simulink model "os_polyphase_synth_4096c"  (R2021a) (continued)
== system os_polyphase_synth_4096c/pfb_fir/pol1_in1_tap23 == (continued)
      | C-C-B*(D+A)
      | C-C-B*(D+A)-CARRYIN
      | C-C-B*(D+ACIN)
      | C-C-B*(D+ACIN)-CARRYIN
      | C-C-B*(D-A)
      | C-C-B*(D-A)-CARRYIN
      | C-C-B*(D-ACIN)
      | C-C-B*(D-ACIN)-CARRYIN
      | C-C-B*A
      | C-C-B*A-CARRYIN
      | C-C-B*ACIN
      | C-C-B*ACIN-CARRYIN
      | C-C-B*D
      | C-C-B*D-CARRYIN
      | C-C-BCIN*(A+D)
      | C-C-BCIN*(A+D)-CARRYIN
      | C-C-BCIN*(ACIN+D)
      | C-C-BCIN*(ACIN+D)-CARRYIN
      | C-C-BCIN*(D+A)
      | C-C-BCIN*(D+A)-CARRYIN
      | C-C-BCIN*(D+ACIN)
      | C-C-BCIN*(D+ACIN)-CARRYIN
      | C-C-BCIN*(D-A)
      | C-C-BCIN*(D-A)-CARRYIN
      | C-C-BCIN*(D-ACIN)
      | C-C-BCIN*(D-ACIN)-CARRYIN
      | C-C-BCIN*A
      | C-C-BCIN*A-CARRYIN
      | C-C-BCIN*ACIN
      | C-C-BCIN*ACIN-CARRYIN
      | C-C-BCIN*D
      | C-C-BCIN*D-CARRYIN
      | C-C-C
      | C-C-C-CARRYIN
      | C-C-C-CONCAT
      | C-C-C-CONCAT-CARRYIN
      | C-C-C-P
      | C-C-C-P-CARRYIN
      | C-C-CARRYCASCIN
      | C-C-CARRYIN
      | C-C-CONCAT
      | C-C-CONCAT-C
      | C-C-CONCAT-C-CARRYIN
      | C-C-CONCAT-CARRYIN
      | C-C-CONCAT-P
      | C-C-CONCAT-P-CARRYIN
      | C-C-D
      | C-C-D*B
      | C-C-D*B-CARRYIN
      | C-C-D*BCIN
      | C-C-D*BCIN-CARRYIN
      | C-C-D*D
      | C-C-D*D-CARRYIN
      | C-C-D-CARRYIN
      | C-C-P
      | C-C-P-C
      | C-C-P-C-CARRYIN
      | C-C-P-CARRYIN
      | C-C-P-CONCAT
      | C-C-P-CONCAT-CARRYIN
      | C-C-P-P
      | C-C-P-P-CARRYIN
      | C-CARRYCASCIN
      | C-CARRYIN
      | C-CONCAT
      | C-CONCAT-C
      | C-CONCAT-C-C
      | C-CONCAT-C-C-CARRYIN
      | C-CONCAT-C-CARRYIN
      | C-CONCAT-C-P
      | C-CONCAT-C-P-CARRYIN
      | C-CONCAT-CARRYCASCIN
      | C-CONCAT-CARRYIN
      | C-CONCAT-P
      | C-CONCAT-P-C
      | C-CONCAT-P-C-CARRYIN
      | C-CONCAT-P-CARRYIN
      | C-D
      | C-D*B
      | C-D*B-C
      | C-D*B-C-CARRYIN
      | C-D*B-CARRYIN
      | C-D*B-P
      | C-D*B-P-CARRYIN
      | C-D*BCIN
      | C-D*BCIN-C
      | C-D*BCIN-C-CARRYIN
      | C-D*BCIN-CARRYIN
      | C-D*BCIN-P
      | C-D*BCIN-P-CARRYIN
      | C-D*D
      | C-D*D-C
      | C-D*D-C-CARRYIN
      | C-D*D-CARRYIN
      | C-D*D-P
      | C-D*D-P-CARRYIN
      | C-D-C
      | C-D-C-CARRYIN
      | C-D-CARRYIN
      | C-D-P
      | C-D-P-CARRYIN
      | C-P
      | C-P-(A+D)
      | C-P-(A+D)*(A+D)
      | C-P-(A+D)*(A+D)-CARRYIN
      | C-P-(A+D)*B
      | C-P-(A+D)*B-CARRYIN
      | C-P-(A+D)*BCIN
      | C-P-(A+D)*BCIN-CARRYIN
      | C-P-(A+D)-CARRYIN
      | C-P-(ACIN+D)
      | C-P-(ACIN+D)*(ACIN+D)
      | C-P-(ACIN+D)*(ACIN+D)-CARRYIN
      | C-P-(ACIN+D)*B
      | C-P-(ACIN+D)*B-CARRYIN
      | C-P-(ACIN+D)*BCIN
      | C-P-(ACIN+D)*BCIN-CARRYIN
      | C-P-(ACIN+D)-CARRYIN
      | C-P-(D+A)
      | C-P-(D+A)*(D+A)
      | C-P-(D+A)*(D+A)-CARRYIN
      | C-P-(D+A)*B
      | C-P-(D+A)*B-CARRYIN
      | C-P-(D+A)*BCIN
      | C-P-(D+A)*BCIN-CARRYIN
      | C-P-(D+A)-CARRYIN
      | C-P-(D+ACIN)
      | C-P-(D+ACIN)*(D+ACIN)
      | C-P-(D+ACIN)*(D+ACIN)-CARRYIN
      | C-P-(D+ACIN)*B
      | C-P-(D+ACIN)*B-CARRYIN
      | C-P-(D+ACIN)*BCIN
      | C-P-(D+ACIN)*BCIN-CARRYIN
      | C-P-(D+ACIN)-CARRYIN
      | C-P-(D-A)
      | C-P-(D-A)*(D-A)
      | C-P-(D-A)*(D-A)-CARRYIN
      | C-P-(D-A)*B
      | C-P-(D-A)*B-CARRYIN
      | C-P-(D-A)*BCIN
      | C-P-(D-A)*BCIN-CARRYIN
      | C-P-(D-A)-CARRYIN
      | C-P-(D-ACIN)
      | C-P-(D-ACIN)*(D-ACIN)
      | C-P-(D-ACIN)*(D-ACIN)-CARRYIN
      | C-P-(D-ACIN)*B
      | C-P-(D-ACIN)*B-CARRYIN
      | C-P-(D-ACIN)*BCIN
      | C-P-(D-ACIN)*BCIN-CARRYIN
      | C-P-(D-ACIN)-CARRYIN
      | C-P-A
      | C-P-A*A
      | C-P-A*A-CARRYIN
      | C-P-A*B
      | C-P-A*B-CARRYIN
      | C-P-A*BCIN
      | C-P-A*BCIN-CARRYIN
      | C-P-A-CARRYIN
      | C-P-ACIN
      | C-P-ACIN*ACIN
      | C-P-ACIN*ACIN-CARRYIN
      | C-P-ACIN*B
      | C-P-ACIN*B-CARRYIN
      | C-P-ACIN*BCIN
      | C-P-ACIN*BCIN-CARRYIN
      | C-P-ACIN-CARRYIN
      | C-P-B*(A+D)
      | C-P-B*(A+D)-CARRYIN
      | C-P-B*(ACIN+D)
      | C-P-B*(ACIN+D)-CARRYIN
      | C-P-B*(D+A)
      | C-P-B*(D+A)-CARRYIN
      | C-P-B*(D+ACIN)
      | C-P-B*(D+ACIN)-CARRYIN
      | C-P-B*(D-A)
      | C-P-B*(D-A)-CARRYIN
      | C-P-B*(D-ACIN)
      | C-P-B*(D-ACIN)-CARRYIN
      | C-P-B*A
      | C-P-B*A-CARRYIN
      | C-P-B*ACIN
      | C-P-B*ACIN-CARRYIN
      | C-P-B*D
      | C-P-B*D-CARRYIN
      | C-P-BCIN*(A+D)
      | C-P-BCIN*(A+D)-CARRYIN
      | C-P-BCIN*(ACIN+D)
      | C-P-BCIN*(ACIN+D)-CARRYIN
      | C-P-BCIN*(D+A)
      | C-P-BCIN*(D+A)-CARRYIN
      | C-P-BCIN*(D+ACIN)
      | C-P-BCIN*(D+ACIN)-CARRYIN
      | C-P-BCIN*(D-A)
      | C-P-BCIN*(D-A)-CARRYIN
      | C-P-BCIN*(D-ACIN)
      | C-P-BCIN*(D-ACIN)-CARRYIN
      | C-P-BCIN*A
      | C-P-BCIN*A-CARRYIN
      | C-P-BCIN*ACIN
      | C-P-BCIN*ACIN-CARRYIN
      | C-P-BCIN*D
      | C-P-BCIN*D-CARRYIN
      | C-P-C
      | C-P-C-C
      | C-P-C-C-CARRYIN
      | C-P-C-CARRYIN
      | C-P-C-CONCAT
      | C-P-C-CONCAT-CARRYIN
      | C-P-C-P
      | C-P-C-P-CARRYIN
      | C-P-CARRYCASCIN
      | C-P-CARRYIN
      | C-P-CONCAT
      | C-P-CONCAT-C
      | C-P-CONCAT-C-CARRYIN
      | C-P-CONCAT-CARRYIN
      | C-P-D
      | C-P-D*B
      | C-P-D*B-CARRYIN
      | C-P-D*BCIN
      | C-P-D*BCIN-CARRYIN
      | C-P-D*D
      | C-P-D*D-CARRYIN
      | C-P-D-CARRYIN
      | C-P-P
      | C-P-P-C
      | C-P-P-C-CARRYIN
      | C-P-P-CARRYIN
      | C-P>>17
      | C-PCIN
      | C-PCIN>>17
      | CARRYIN
      | CONCAT
      | CONCAT+C
      | CONCAT+C+C
      | CONCAT+C+C+C
      | CONCAT+C+C+C+CARRYIN
      | CONCAT+C+C+CARRYIN
      | CONCAT+C+C+P
      | CONCAT+C+C+P+CARRYIN
      | CONCAT+C+C+P>>17
      | CONCAT+C+C+P>>17+CARRYIN
      | CONCAT+C+C+PCIN
      | CONCAT+C+C+PCIN+CARRYIN
      | CONCAT+C+C+PCIN>>17
      | CONCAT+C+C+PCIN>>17+CARRYIN
      | CONCAT+C+C-C
      | CONCAT+C+C-P
      | CONCAT+C+C-P>>17
      | CONCAT+C+C-PCIN
      | CONCAT+C+C-PCIN>>17
      | CONCAT+C+CARRYCASCIN
      | CONCAT+C+CARRYIN
      | CONCAT+C+P
      | CONCAT+C+P+C
      | CONCAT+C+P+C+CARRYIN
      | CONCAT+C+P+CARRYIN
      | CONCAT+C+P+P
      | CONCAT+C+P+P+CARRYIN
      | CONCAT+C+P+P>>17
      | CONCAT+C+P+P>>17+CARRYIN
      | CONCAT+C+P+PCIN
      | CONCAT+C+P+PCIN+CARRYIN
      | CONCAT+C+P+PCIN>>17
      | CONCAT+C+P+PCIN>>17+CARRYIN
      | CONCAT+C+P-C
      | CONCAT+C+P-P
      | CONCAT+C+P-P>>17
      | CONCAT+C+P-PCIN
      | CONCAT+C+P-PCIN>>17
      | CONCAT+C+P>>17
      | CONCAT+C+P>>17+C
      | CONCAT+C+P>>17+C+CARRYIN
      | CONCAT+C+P>>17+CARRYIN
      | CONCAT+C+P>>17+P
      | CONCAT+C+P>>17+P+CARRYIN
      | CONCAT+C+PCIN
      | CONCAT+C+PCIN+C
      | CONCAT+C+PCIN+C+CARRYIN
      | CONCAT+C+PCIN+CARRYIN
      | CONCAT+C+PCIN+P
      | CONCAT+C+PCIN+P+CARRYIN
      | CONCAT+C+PCIN>>17
      | CONCAT+C+PCIN>>17+C
      | CONCAT+C+PCIN>>17+C+CARRYIN
      | CONCAT+C+PCIN>>17+CARRYIN
      | CONCAT+C+PCIN>>17+P
      | CONCAT+C+PCIN>>17+P+CARRYIN
      | CONCAT+C-C
      | CONCAT+C-P
      | CONCAT+C-P>>17
      | CONCAT+C-PCIN
      | CONCAT+C-PCIN>>17
      | CONCAT+CARRYCASCIN
      | CONCAT+CARRYIN
      | CONCAT+P
      | CONCAT+P+C
      | CONCAT+P+C+C
      | CONCAT+P+C+C+CARRYIN
      | CONCAT+P+C+CARRYIN
      | CONCAT+P+C+P
      | CONCAT+P+C+P+CARRYIN
      | CONCAT+P+C+P>>17
      | CONCAT+P+C+P>>17+CARRYIN
      | CONCAT+P+C+PCIN
      | CONCAT+P+C+PCIN+CARRYIN
      | CONCAT+P+C+PCIN>>17
      | CONCAT+P+C+PCIN>>17+CARRYIN
      | CONCAT+P+C-C
      | CONCAT+P+C-P
      | CONCAT+P+C-P>>17
      | CONCAT+P+C-PCIN
      | CONCAT+P+C-PCIN>>17
      | CONCAT+P+CARRYCASCIN
      | CONCAT+P+CARRYIN
      | CONCAT+P+P
      | CONCAT+P+P+C
      | CONCAT+P+P+C+CARRYIN
      | CONCAT+P+P+CARRYIN
      | CONCAT+P+P>>17
      | CONCAT+P+P>>17+C
      | CONCAT+P+P>>17+C+CARRYIN
      | CONCAT+P+P>>17+CARRYIN
      | CONCAT+P+PCIN
      | CONCAT+P+PCIN+C
      | CONCAT+P+PCIN+C+CARRYIN
      | CONCAT+P+PCIN+CARRYIN
      | CONCAT+P+PCIN>>17
      | CONCAT+P+PCIN>>17+C
      | CONCAT+P+PCIN>>17+C+CARRYIN
      | CONCAT+P+PCIN>>17+CARRYIN
      | CONCAT+P-C
      | CONCAT+P-P
      | CONCAT+P-P>>17
      | CONCAT+P-PCIN
      | CONCAT+P-PCIN>>17
      | CONCAT+P>>17
      | CONCAT+P>>17+C
      | CONCAT+P>>17+C+C
      | CONCAT+P>>17+C+C+CARRYIN
      | CONCAT+P>>17+C+CARRYIN
      | CONCAT+P>>17+C+P
      | CONCAT+P>>17+C+P+CARRYIN
      | CONCAT+P>>17+CARRYIN
      | CONCAT+P>>17+P
      | CONCAT+P>>17+P+C
      | CONCAT+P>>17+P+C+CARRYIN
      | CONCAT+P>>17+P+CARRYIN
      | CONCAT+PCIN
      | CONCAT+PCIN+C
      | CONCAT+PCIN+C+C
      | CONCAT+PCIN+C+C+CARRYIN
      | CONCAT+PCIN+C+CARRYIN
      | CONCAT+PCIN+C+P
      | CONCAT+PCIN+C+P+CARRYIN
      | CONCAT+PCIN+CARRYIN
      | CONCAT+PCIN+P
      | CONCAT+PCIN+P+C
      | CONCAT+PCIN+P+C+CARRYIN
      | CONCAT+PCIN+P+CARRYIN
      | CONCAT+PCIN>>17
      | CONCAT+PCIN>>17+C
      | CONCAT+PCIN>>17+C+C
      | CONCAT+PCIN>>17+C+C+CARRYIN
      | CONCAT+PCIN>>17+C+CARRYIN
      | CONCAT+PCIN>>17+C+P
      | CONCAT+PCIN>>17+C+P+CARRYIN
      | CONCAT+PCIN>>17+CARRYIN
      | CONCAT+PCIN>>17+P
      | CONCAT+PCIN>>17+P+C
      | CONCAT+PCIN>>17+P+C+CARRYIN
      | CONCAT+PCIN>>17+P+CARRYIN
      | CONCAT-C
      | CONCAT-P
      | CONCAT-P>>17
      | CONCAT-PCIN
      | CONCAT-PCIN>>17
      | D
      | D*B
      | D*B+C
      | D*B+C+C
      | D*B+C+C+CARRYIN
      | D*B+C+CARRYIN
      | D*B+C+P
      | D*B+C+P+CARRYIN
      | D*B+C+P>>17
      | D*B+C+P>>17+CARRYIN
      | D*B+C+PCIN
      | D*B+C+PCIN+CARRYIN
      | D*B+C+PCIN>>17
      | D*B+C+PCIN>>17+CARRYIN
      | D*B+C-C
      | D*B+C-P
      | D*B+C-P>>17
      | D*B+C-PCIN
      | D*B+C-PCIN>>17
      | D*B+CARRYIN
      | D*B+P
      | D*B+P+C
      | D*B+P+C+CARRYIN
      | D*B+P+CARRYIN
      | D*B+P+P
      | D*B+P+P+CARRYIN
      | D*B+P+P>>17
      | D*B+P+P>>17+CARRYIN
      | D*B+P+PCIN
      | D*B+P+PCIN+CARRYIN
      | D*B+P+PCIN>>17
      | D*B+P+PCIN>>17+CARRYIN
      | D*B+P-C
      | D*B+P-P
      | D*B+P-P>>17
      | D*B+P-PCIN
      | D*B+P-PCIN>>17
      | D*B+P>>17
      | D*B+P>>17+C
      | D*B+P>>17+C+CARRYIN
      | D*B+P>>17+CARRYIN
      | D*B+P>>17+P
      | D*B+P>>17+P+CARRYIN
      | D*B+PCIN
      | D*B+PCIN+C
      | D*B+PCIN+C+CARRYIN
      | D*B+PCIN+CARRYIN
      | D*B+PCIN+P
      | D*B+PCIN+P+CARRYIN
      | D*B+PCIN>>17
      | D*B+PCIN>>17+C
      | D*B+PCIN>>17+C+CARRYIN
      | D*B+PCIN>>17+CARRYIN
      | D*B+PCIN>>17+P
      | D*B+PCIN>>17+P+CARRYIN
      | D*B-C
      | D*B-P
      | D*B-P>>17
      | D*B-PCIN
      | D*B-PCIN>>17
      | D*BCIN
      | D*BCIN+C
      | D*BCIN+C+C
      | D*BCIN+C+C+CARRYIN
      | D*BCIN+C+CARRYIN
      | D*BCIN+C+P
      | D*BCIN+C+P+CARRYIN
      | D*BCIN+C+P>>17
      | D*BCIN+C+P>>17+CARRYIN
      | D*BCIN+C+PCIN
      | D*BCIN+C+PCIN+CARRYIN
      | D*BCIN+C+PCIN>>17
      | D*BCIN+C+PCIN>>17+CARRYIN
      | D*BCIN+C-C
      | D*BCIN+C-P
      | D*BCIN+C-P>>17
      | D*BCIN+C-PCIN
      | D*BCIN+C-PCIN>>17
      | D*BCIN+CARRYIN
      | D*BCIN+P
      | D*BCIN+P+C
      | D*BCIN+P+C+CARRYIN
      | D*BCIN+P+CARRYIN
      | D*BCIN+P+P
      | D*BCIN+P+P+CARRYIN
      | D*BCIN+P+P>>17
      | D*BCIN+P+P>>17+CARRYIN
      | D*BCIN+P+PCIN
      | D*BCIN+P+PCIN+CARRYIN
      | D*BCIN+P+PCIN>>17
      | D*BCIN+P+PCIN>>17+CARRYIN
      | D*BCIN+P-C
      | D*BCIN+P-P
      | D*BCIN+P-P>>17
      | D*BCIN+P-PCIN
      | D*BCIN+P-PCIN>>17
      | D*BCIN+P>>17
      | D*BCIN+P>>17+C
      | D*BCIN+P>>17+C+CARRYIN
      | D*BCIN+P>>17+CARRYIN
      | D*BCIN+P>>17+P
      | D*BCIN+P>>17+P+CARRYIN
      | D*BCIN+PCIN
      | D*BCIN+PCIN+C
      | D*BCIN+PCIN+C+CARRYIN
      | D*BCIN+PCIN+CARRYIN
      | D*BCIN+PCIN+P
      | D*BCIN+PCIN+P+CARRYIN
      | D*BCIN+PCIN>>17
      | D*BCIN+PCIN>>17+C
      | D*BCIN+PCIN>>17+C+CARRYIN
      | D*BCIN+PCIN>>17+CARRYIN
      | D*BCIN+PCIN>>17+P
      | D*BCIN+PCIN>>17+P+CARRYIN
      | D*BCIN-C
      | D*BCIN-P
      | D*BCIN-P>>17
      | D*BCIN-PCIN
      | D*BCIN-PCIN>>17
      | D*D
      | D*D+C
      | D*D+C+C
      | D*D+C+C+CARRYIN
      | D*D+C+CARRYIN
      | D*D+C+P
      | D*D+C+P+CARRYIN
      | D*D+C+P>>17
      | D*D+C+P>>17+CARRYIN
      | D*D+C+PCIN
      | D*D+C+PCIN+CARRYIN
      | D*D+C+PCIN>>17
      | D*D+C+PCIN>>17+CARRYIN
      | D*D+C-C
      | D*D+C-P
      | D*D+C-P>>17
      | D*D+C-PCIN
      | D*D+C-PCIN>>17
      | D*D+CARRYIN
      | D*D+P
      | D*D+P+C
      | D*D+P+C+CARRYIN
      | D*D+P+CARRYIN
      | D*D+P+P
      | D*D+P+P+CARRYIN
      | D*D+P+P>>17
      | D*D+P+P>>17+CARRYIN
      | D*D+P+PCIN
      | D*D+P+PCIN+CARRYIN
      | D*D+P+PCIN>>17
      | D*D+P+PCIN>>17+CARRYIN
      | D*D+P-C
      | D*D+P-P
      | D*D+P-P>>17
      | D*D+P-PCIN
      | D*D+P-PCIN>>17
      | D*D+P>>17
      | D*D+P>>17+C
      | D*D+P>>17+C+CARRYIN
      | D*D+P>>17+CARRYIN
      | D*D+P>>17+P
      | D*D+P>>17+P+CARRYIN
      | D*D+PCIN
      | D*D+PCIN+C
      | D*D+PCIN+C+CARRYIN
      | D*D+PCIN+CARRYIN
      | D*D+PCIN+P
      | D*D+PCIN+P+CARRYIN
      | D*D+PCIN>>17
      | D*D+PCIN>>17+C
      | D*D+PCIN>>17+C+CARRYIN
      | D*D+PCIN>>17+CARRYIN
      | D*D+PCIN>>17+P
      | D*D+PCIN>>17+P+CARRYIN
      | D*D-C
      | D*D-P
      | D*D-P>>17
      | D*D-PCIN
      | D*D-PCIN>>17
      | D+C
      | D+C+C
      | D+C+C+CARRYIN
      | D+C+CARRYIN
      | D+C+P
      | D+C+P+CARRYIN
      | D+C+P>>17
      | D+C+P>>17+CARRYIN
      | D+C+PCIN
      | D+C+PCIN+CARRYIN
      | D+C+PCIN>>17
      | D+C+PCIN>>17+CARRYIN
      | D+C-C
      | D+C-P
      | D+C-P>>17
      | D+C-PCIN
      | D+C-PCIN>>17
      | D+CARRYIN
      | D+P
      | D+P+C
      | D+P+C+CARRYIN
      | D+P+CARRYIN
      | D+P+P
      | D+P+P+CARRYIN
      | D+P+P>>17
      | D+P+P>>17+CARRYIN
      | D+P+PCIN
      | D+P+PCIN+CARRYIN
      | D+P+PCIN>>17
      | D+P+PCIN>>17+CARRYIN
      | D+P-C
      | D+P-P
      | D+P-P>>17
      | D+P-PCIN
      | D+P-PCIN>>17
      | D+P>>17
      | D+P>>17+C
      | D+P>>17+C+CARRYIN
      | D+P>>17+CARRYIN
      | D+P>>17+P
      | D+P>>17+P+CARRYIN
      | D+PCIN
      | D+PCIN+C
      | D+PCIN+C+CARRYIN
      | D+PCIN+CARRYIN
      | D+PCIN+P
      | D+PCIN+P+CARRYIN
      | D+PCIN>>17
      | D+PCIN>>17+C
      | D+PCIN>>17+C+CARRYIN
      | D+PCIN>>17+CARRYIN
      | D+PCIN>>17+P
      | D+PCIN>>17+P+CARRYIN
      | D-C
      | D-P
      | D-P>>17
      | D-PCIN
      | D-PCIN>>17
      | P
      | P+(A+D)
      | P+(A+D)*(A+D)
      | P+(A+D)*(A+D)+C
      | P+(A+D)*(A+D)+C+CARRYIN
      | P+(A+D)*(A+D)+CARRYIN
      | P+(A+D)*(A+D)+P
      | P+(A+D)*(A+D)+P+CARRYIN
      | P+(A+D)*(A+D)+P>>17
      | P+(A+D)*(A+D)+P>>17+CARRYIN
      | P+(A+D)*(A+D)+PCIN
      | P+(A+D)*(A+D)+PCIN+CARRYIN
      | P+(A+D)*(A+D)+PCIN>>17
      | P+(A+D)*(A+D)+PCIN>>17+CARRYIN
      | P+(A+D)*(A+D)-C
      | P+(A+D)*(A+D)-P
      | P+(A+D)*(A+D)-P>>17
      | P+(A+D)*(A+D)-PCIN
      | P+(A+D)*(A+D)-PCIN>>17
      | P+(A+D)*B
      | P+(A+D)*B+C
      | P+(A+D)*B+C+CARRYIN
      | P+(A+D)*B+CARRYIN
      | P+(A+D)*B+P
      | P+(A+D)*B+P+CARRYIN
      | P+(A+D)*B+P>>17
      | P+(A+D)*B+P>>17+CARRYIN
      | P+(A+D)*B+PCIN
      | P+(A+D)*B+PCIN+CARRYIN
      | P+(A+D)*B+PCIN>>17
      | P+(A+D)*B+PCIN>>17+CARRYIN
      | P+(A+D)*B-C
      | P+(A+D)*B-P
      | P+(A+D)*B-P>>17
      | P+(A+D)*B-PCIN
      | P+(A+D)*B-PCIN>>17
      | P+(A+D)*BCIN
      | P+(A+D)*BCIN+C
      | P+(A+D)*BCIN+C+CARRYIN
      | P+(A+D)*BCIN+CARRYIN
      | P+(A+D)*BCIN+P
      | P+(A+D)*BCIN+P+CARRYIN
      | P+(A+D)*BCIN+P>>17
      | P+(A+D)*BCIN+P>>17+CARRYIN
      | P+(A+D)*BCIN+PCIN
      | P+(A+D)*BCIN+PCIN+CARRYIN
      | P+(A+D)*BCIN+PCIN>>17
      | P+(A+D)*BCIN+PCIN>>17+CARRYIN
      | P+(A+D)*BCIN-C
      | P+(A+D)*BCIN-P
      | P+(A+D)*BCIN-P>>17
      | P+(A+D)*BCIN-PCIN
      | P+(A+D)*BCIN-PCIN>>17
      | P+(A+D)+C
      | P+(A+D)+C+CARRYIN
      | P+(A+D)+CARRYIN
      | P+(A+D)+P
      | P+(A+D)+P+CARRYIN
      | P+(A+D)+P>>17
      | P+(A+D)+P>>17+CARRYIN
      | P+(A+D)+PCIN
      | P+(A+D)+PCIN+CARRYIN
      | P+(A+D)+PCIN>>17
      | P+(A+D)+PCIN>>17+CARRYIN
      | P+(A+D)-C
      | P+(A+D)-P
      | P+(A+D)-P>>17
      | P+(A+D)-PCIN
      | P+(A+D)-PCIN>>17
      | P+(ACIN+D)
      | P+(ACIN+D)*(ACIN+D)
      | P+(ACIN+D)*(ACIN+D)+C
      | P+(ACIN+D)*(ACIN+D)+C+CARRYIN
      | P+(ACIN+D)*(ACIN+D)+CARRYIN
      | P+(ACIN+D)*(ACIN+D)+P
      | P+(ACIN+D)*(ACIN+D)+P+CARRYIN
      | P+(ACIN+D)*(ACIN+D)+P>>17
      | P+(ACIN+D)*(ACIN+D)+P>>17+CARRYIN
      | P+(ACIN+D)*(ACIN+D)+PCIN
      | P+(ACIN+D)*(ACIN+D)+PCIN+CARRYIN
      | P+(ACIN+D)*(ACIN+D)+PCIN>>17
      | P+(ACIN+D)*(ACIN+D)+PCIN>>17+CARRYIN
      | P+(ACIN+D)*(ACIN+D)-C
      | P+(ACIN+D)*(ACIN+D)-P
      | P+(ACIN+D)*(ACIN+D)-P>>17
      | P+(ACIN+D)*(ACIN+D)-PCIN
      | P+(ACIN+D)*(ACIN+D)-PCIN>>17
      | P+(ACIN+D)*B
      | P+(ACIN+D)*B+C
      | P+(ACIN+D)*B+C+CARRYIN
      | P+(ACIN+D)*B+CARRYIN
      | P+(ACIN+D)*B+P
      | P+(ACIN+D)*B+P+CARRYIN
      | P+(ACIN+D)*B+P>>17
      | P+(ACIN+D)*B+P>>17+CARRYIN
      | P+(ACIN+D)*B+PCIN
      | P+(ACIN+D)*B+PCIN+CARRYIN
      | P+(ACIN+D)*B+PCIN>>17
      | P+(ACIN+D)*B+PCIN>>17+CARRYIN
      | P+(ACIN+D)*B-C
      | P+(ACIN+D)*B-P
      | P+(ACIN+D)*B-P>>17
      | P+(ACIN+D)*B-PCIN
      | P+(ACIN+D)*B-PCIN>>17
      | P+(ACIN+D)*BCIN
      | P+(ACIN+D)*BCIN+C
      | P+(ACIN+D)*BCIN+C+CARRYIN
      | P+(ACIN+D)*BCIN+CARRYIN
      | P+(ACIN+D)*BCIN+P
      | P+(ACIN+D)*BCIN+P+CARRYIN
      | P+(ACIN+D)*BCIN+P>>17
      | P+(ACIN+D)*BCIN+P>>17+CARRYIN
      | P+(ACIN+D)*BCIN+PCIN
      | P+(ACIN+D)*BCIN+PCIN+CARRYIN
      | P+(ACIN+D)*BCIN+PCIN>>17
      | P+(ACIN+D)*BCIN+PCIN>>17+CARRYIN
      | P+(ACIN+D)*BCIN-C
      | P+(ACIN+D)*BCIN-P
      | P+(ACIN+D)*BCIN-P>>17
      | P+(ACIN+D)*BCIN-PCIN
      | P+(ACIN+D)*BCIN-PCIN>>17
      | P+(ACIN+D)+C
      | P+(ACIN+D)+C+CARRYIN
      | P+(ACIN+D)+CARRYIN
      | P+(ACIN+D)+P
      | P+(ACIN+D)+P+CARRYIN
      | P+(ACIN+D)+P>>17
      | P+(ACIN+D)+P>>17+CARRYIN
      | P+(ACIN+D)+PCIN
      | P+(ACIN+D)+PCIN+CARRYIN
      | P+(ACIN+D)+PCIN>>17
      | P+(ACIN+D)+PCIN>>17+CARRYIN
      | P+(ACIN+D)-C
      | P+(ACIN+D)-P
      | P+(ACIN+D)-P>>17
      | P+(ACIN+D)-PCIN
      | P+(ACIN+D)-PCIN>>17
      | P+(D+A)
      | P+(D+A)*(D+A)
      | P+(D+A)*(D+A)+C
      | P+(D+A)*(D+A)+C+CARRYIN
      | P+(D+A)*(D+A)+CARRYIN
      | P+(D+A)*(D+A)+P
      | P+(D+A)*(D+A)+P+CARRYIN
      | P+(D+A)*(D+A)+P>>17
      | P+(D+A)*(D+A)+P>>17+CARRYIN
      | P+(D+A)*(D+A)+PCIN
      | P+(D+A)*(D+A)+PCIN+CARRYIN
      | P+(D+A)*(D+A)+PCIN>>17
      | P+(D+A)*(D+A)+PCIN>>17+CARRYIN
      | P+(D+A)*(D+A)-C
      | P+(D+A)*(D+A)-P
      | P+(D+A)*(D+A)-P>>17
      | P+(D+A)*(D+A)-PCIN
      | P+(D+A)*(D+A)-PCIN>>17
      | P+(D+A)*B
      | P+(D+A)*B+C
      | P+(D+A)*B+C+CARRYIN
      | P+(D+A)*B+CARRYIN
      | P+(D+A)*B+P
      | P+(D+A)*B+P+CARRYIN
      | P+(D+A)*B+P>>17
      | P+(D+A)*B+P>>17+CARRYIN
      | P+(D+A)*B+PCIN
      | P+(D+A)*B+PCIN+CARRYIN
      | P+(D+A)*B+PCIN>>17
      | P+(D+A)*B+PCIN>>17+CARRYIN
      | P+(D+A)*B-C
      | P+(D+A)*B-P
      | P+(D+A)*B-P>>17
      | P+(D+A)*B-PCIN
      | P+(D+A)*B-PCIN>>17
      | P+(D+A)*BCIN
      | P+(D+A)*BCIN+C
      | P+(D+A)*BCIN+C+CARRYIN
      | P+(D+A)*BCIN+CARRYIN
      | P+(D+A)*BCIN+P
      | P+(D+A)*BCIN+P+CARRYIN
      | P+(D+A)*BCIN+P>>17
      | P+(D+A)*BCIN+P>>17+CARRYIN
      | P+(D+A)*BCIN+PCIN
      | P+(D+A)*BCIN+PCIN+CARRYIN
      | P+(D+A)*BCIN+PCIN>>17
      | P+(D+A)*BCIN+PCIN>>17+CARRYIN
      | P+(D+A)*BCIN-C
      | P+(D+A)*BCIN-P
      | P+(D+A)*BCIN-P>>17
      | P+(D+A)*BCIN-PCIN
      | P+(D+A)*BCIN-PCIN>>17
      | P+(D+A)+C
      | P+(D+A)+C+CARRYIN
      | P+(D+A)+CARRYIN
      | P+(D+A)+P
      | P+(D+A)+P+CARRYIN
      | P+(D+A)+P>>17
      | P+(D+A)+P>>17+CARRYIN
      | P+(D+A)+PCIN
      | P+(D+A)+PCIN+CARRYIN
      | P+(D+A)+PCIN>>17
      | P+(D+A)+PCIN>>17+CARRYIN
      | P+(D+A)-C
      | P+(D+A)-P
      | P+(D+A)-P>>17
      | P+(D+A)-PCIN
      | P+(D+A)-PCIN>>17
      | P+(D+ACIN)
      | P+(D+ACIN)*(D+ACIN)
      | P+(D+ACIN)*(D+ACIN)+C
      | P+(D+ACIN)*(D+ACIN)+C+CARRYIN
      | P+(D+ACIN)*(D+ACIN)+CARRYIN
      | P+(D+ACIN)*(D+ACIN)+P
      | P+(D+ACIN)*(D+ACIN)+P+CARRYIN
      | P+(D+ACIN)*(D+ACIN)+P>>17
      | P+(D+ACIN)*(D+ACIN)+P>>17+CARRYIN
      | P+(D+ACIN)*(D+ACIN)+PCIN
      | P+(D+ACIN)*(D+ACIN)+PCIN+CARRYIN
      | P+(D+ACIN)*(D+ACIN)+PCIN>>17
      | P+(D+ACIN)*(D+ACIN)+PCIN>>17+CARRYIN
      | P+(D+ACIN)*(D+ACIN)-C
      | P+(D+ACIN)*(D+ACIN)-P
      | P+(D+ACIN)*(D+ACIN)-P>>17
      | P+(D+ACIN)*(D+ACIN)-PCIN
      | P+(D+ACIN)*(D+ACIN)-PCIN>>17
      | P+(D+ACIN)*B
      | P+(D+ACIN)*B+C
      | P+(D+ACIN)*B+C+CARRYIN
      | P+(D+ACIN)*B+CARRYIN
      | P+(D+ACIN)*B+P
      | P+(D+ACIN)*B+P+CARRYIN
      | P+(D+ACIN)*B+P>>17
      | P+(D+ACIN)*B+P>>17+CARRYIN
      | P+(D+ACIN)*B+PCIN
      | P+(D+ACIN)*B+PCIN+CARRYIN
      | P+(D+ACIN)*B+PCIN>>17
      | P+(D+ACIN)*B+PCIN>>17+CARRYIN
      | P+(D+ACIN)*B-C
      | P+(D+ACIN)*B-P
      | P+(D+ACIN)*B-P>>17
      | P+(D+ACIN)*B-PCIN
      | P+(D+ACIN)*B-PCIN>>17
      | P+(D+ACIN)*BCIN
      | P+(D+ACIN)*BCIN+C
      | P+(D+ACIN)*BCIN+C+CARRYIN
      | P+(D+ACIN)*BCIN+CARRYIN
      | P+(D+ACIN)*BCIN+P
      | P+(D+ACIN)*BCIN+P+CARRYIN
      | P+(D+ACIN)*BCIN+P>>17
      | P+(D+ACIN)*BCIN+P>>17+CARRYIN
      | P+(D+ACIN)*BCIN+PCIN
      | P+(D+ACIN)*BCIN+PCIN+CARRYIN
      | P+(D+ACIN)*BCIN+PCIN>>17
      | P+(D+ACIN)*BCIN+PCIN>>17+CARRYIN
      | P+(D+ACIN)*BCIN-C
      | P+(D+ACIN)*BCIN-P
      | P+(D+ACIN)*BCIN-P>>17
      | P+(D+ACIN)*BCIN-PCIN
      | P+(D+ACIN)*BCIN-PCIN>>17
      | P+(D+ACIN)+C
      | P+(D+ACIN)+C+CARRYIN
      | P+(D+ACIN)+CARRYIN
      | P+(D+ACIN)+P
      | P+(D+ACIN)+P+CARRYIN
      | P+(D+ACIN)+P>>17
      | P+(D+ACIN)+P>>17+CARRYIN
      | P+(D+ACIN)+PCIN
      | P+(D+ACIN)+PCIN+CARRYIN
      | P+(D+ACIN)+PCIN>>17
      | P+(D+ACIN)+PCIN>>17+CARRYIN
      | P+(D+ACIN)-C
      | P+(D+ACIN)-P
      | P+(D+ACIN)-P>>17
      | P+(D+ACIN)-PCIN
      | P+(D+ACIN)-PCIN>>17
      | P+(D-A)
      | P+(D-A)*(D-A)
      | P+(D-A)*(D-A)+C
      | P+(D-A)*(D-A)+C+CARRYIN
      | P+(D-A)*(D-A)+CARRYIN
      | P+(D-A)*(D-A)+P
      | P+(D-A)*(D-A)+P+CARRYIN
      | P+(D-A)*(D-A)+P>>17
      | P+(D-A)*(D-A)+P>>17+CARRYIN
      | P+(D-A)*(D-A)+PCIN
      | P+(D-A)*(D-A)+PCIN+CARRYIN
      | P+(D-A)*(D-A)+PCIN>>17
      | P+(D-A)*(D-A)+PCIN>>17+CARRYIN
      | P+(D-A)*(D-A)-C
      | P+(D-A)*(D-A)-P
      | P+(D-A)*(D-A)-P>>17
      | P+(D-A)*(D-A)-PCIN
      | P+(D-A)*(D-A)-PCIN>>17
      | P+(D-A)*B
      | P+(D-A)*B+C
      | P+(D-A)*B+C+CARRYIN
      | P+(D-A)*B+CARRYIN
      | P+(D-A)*B+P
      | P+(D-A)*B+P+CARRYIN
      | P+(D-A)*B+P>>17
      | P+(D-A)*B+P>>17+CARRYIN
      | P+(D-A)*B+PCIN
      | P+(D-A)*B+PCIN+CARRYIN
      | P+(D-A)*B+PCIN>>17
      | P+(D-A)*B+PCIN>>17+CARRYIN
      | P+(D-A)*B-C
      | P+(D-A)*B-P
      | P+(D-A)*B-P>>17
      | P+(D-A)*B-PCIN
      | P+(D-A)*B-PCIN>>17
      | P+(D-A)*BCIN
      | P+(D-A)*BCIN+C
      | P+(D-A)*BCIN+C+CARRYIN
      | P+(D-A)*BCIN+CARRYIN
      | P+(D-A)*BCIN+P
      | P+(D-A)*BCIN+P+CARRYIN
      | P+(D-A)*BCIN+P>>17
      | P+(D-A)*BCIN+P>>17+CARRYIN
      | P+(D-A)*BCIN+PCIN
      | P+(D-A)*BCIN+PCIN+CARRYIN
      | P+(D-A)*BCIN+PCIN>>17
      | P+(D-A)*BCIN+PCIN>>17+CARRYIN
      | P+(D-A)*BCIN-C
      | P+(D-A)*BCIN-P
      | P+(D-A)*BCIN-P>>17
      | P+(D-A)*BCIN-PCIN
      | P+(D-A)*BCIN-PCIN>>17
      | P+(D-A)+C
      | P+(D-A)+C+CARRYIN
      | P+(D-A)+CARRYIN
      | P+(D-A)+P
      | P+(D-A)+P+CARRYIN
      | P+(D-A)+P>>17
      | P+(D-A)+P>>17+CARRYIN
      | P+(D-A)+PCIN
      | P+(D-A)+PCIN+CARRYIN
      | P+(D-A)+PCIN>>17
      | P+(D-A)+PCIN>>17+CARRYIN
      | P+(D-A)-C
      | P+(D-A)-P
      | P+(D-A)-P>>17
      | P+(D-A)-PCIN
      | P+(D-A)-PCIN>>17
      | P+(D-ACIN)
      | P+(D-ACIN)*(D-ACIN)
      | P+(D-ACIN)*(D-ACIN)+C
      | P+(D-ACIN)*(D-ACIN)+C+CARRYIN
      | P+(D-ACIN)*(D-ACIN)+CARRYIN
      | P+(D-ACIN)*(D-ACIN)+P
      | P+(D-ACIN)*(D-ACIN)+P+CARRYIN
      | P+(D-ACIN)*(D-ACIN)+P>>17
      | P+(D-ACIN)*(D-ACIN)+P>>17+CARRYIN
      | P+(D-ACIN)*(D-ACIN)+PCIN
      | P+(D-ACIN)*(D-ACIN)+PCIN+CARRYIN
      | P+(D-ACIN)*(D-ACIN)+PCIN>>17
      | P+(D-ACIN)*(D-ACIN)+PCIN>>17+CARRYIN
      | P+(D-ACIN)*(D-ACIN)-C
      | P+(D-ACIN)*(D-ACIN)-P
      | P+(D-ACIN)*(D-ACIN)-P>>17
      | P+(D-ACIN)*(D-ACIN)-PCIN
      | P+(D-ACIN)*(D-ACIN)-PCIN>>17
      | P+(D-ACIN)*B
      | P+(D-ACIN)*B+C
      | P+(D-ACIN)*B+C+CARRYIN
      | P+(D-ACIN)*B+CARRYIN
      | P+(D-ACIN)*B+P
      | P+(D-ACIN)*B+P+CARRYIN
      | P+(D-ACIN)*B+P>>17
      | P+(D-ACIN)*B+P>>17+CARRYIN
      | P+(D-ACIN)*B+PCIN
      | P+(D-ACIN)*B+PCIN+CARRYIN
      | P+(D-ACIN)*B+PCIN>>17
      | P+(D-ACIN)*B+PCIN>>17+CARRYIN
      | P+(D-ACIN)*B-C
      | P+(D-ACIN)*B-P
      | P+(D-ACIN)*B-P>>17
      | P+(D-ACIN)*B-PCIN
      | P+(D-ACIN)*B-PCIN>>17
      | P+(D-ACIN)*BCIN
      | P+(D-ACIN)*BCIN+C
      | P+(D-ACIN)*BCIN+C+CARRYIN
      | P+(D-ACIN)*BCIN+CARRYIN
      | P+(D-ACIN)*BCIN+P
      | P+(D-ACIN)*BCIN+P+CARRYIN
      | P+(D-ACIN)*BCIN+P>>17
      | P+(D-ACIN)*BCIN+P>>17+CARRYIN
      | P+(D-ACIN)*BCIN+PCIN
      | P+(D-ACIN)*BCIN+PCIN+CARRYIN
      | P+(D-ACIN)*BCIN+PCIN>>17
      | P+(D-ACIN)*BCIN+PCIN>>17+CARRYIN
      | P+(D-ACIN)*BCIN-C
      | P+(D-ACIN)*BCIN-P
      | P+(D-ACIN)*BCIN-P>>17
      | P+(D-ACIN)*BCIN-PCIN
      | P+(D-ACIN)*BCIN-PCIN>>17
      | P+(D-ACIN)+C
      | P+(D-ACIN)+C+CARRYIN
      | P+(D-ACIN)+CARRYIN
      | P+(D-ACIN)+P
      | P+(D-ACIN)+P+CARRYIN
      | P+(D-ACIN)+P>>17
      | P+(D-ACIN)+P>>17+CARRYIN
      | P+(D-ACIN)+PCIN
      | P+(D-ACIN)+PCIN+CARRYIN
      | P+(D-ACIN)+PCIN>>17
      | P+(D-ACIN)+PCIN>>17+CARRYIN
      | P+(D-ACIN)-C
      | P+(D-ACIN)-P
      | P+(D-ACIN)-P>>17
      | P+(D-ACIN)-PCIN
      | P+(D-ACIN)-PCIN>>17
      | P+A
      | P+A*A
      | P+A*A+C
      | P+A*A+C+CARRYIN
      | P+A*A+CARRYIN
      | P+A*A+P
      | P+A*A+P+CARRYIN
      | P+A*A+P>>17
      | P+A*A+P>>17+CARRYIN
      | P+A*A+PCIN
      | P+A*A+PCIN+CARRYIN
      | P+A*A+PCIN>>17
      | P+A*A+PCIN>>17+CARRYIN
      | P+A*A-C
      | P+A*A-P
      | P+A*A-P>>17
      | P+A*A-PCIN
      | P+A*A-PCIN>>17
      | P+A*B
      | P+A*B+C
      | P+A*B+C+CARRYIN
      | P+A*B+CARRYIN
      | P+A*B+P
      | P+A*B+P+CARRYIN
      | P+A*B+P>>17
      | P+A*B+P>>17+CARRYIN
      | P+A*B+PCIN
      | P+A*B+PCIN+CARRYIN
      | P+A*B+PCIN>>17
      | P+A*B+PCIN>>17+CARRYIN
      | P+A*B-C
      | P+A*B-P
      | P+A*B-P>>17
      | P+A*B-PCIN
      | P+A*B-PCIN>>17
      | P+A*BCIN
      | P+A*BCIN+C
      | P+A*BCIN+C+CARRYIN
      | P+A*BCIN+CARRYIN
      | P+A*BCIN+P
      | P+A*BCIN+P+CARRYIN
      | P+A*BCIN+P>>17
      | P+A*BCIN+P>>17+CARRYIN
      | P+A*BCIN+PCIN
      | P+A*BCIN+PCIN+CARRYIN
      | P+A*BCIN+PCIN>>17
      | P+A*BCIN+PCIN>>17+CARRYIN
      | P+A*BCIN-C
      | P+A*BCIN-P
      | P+A*BCIN-P>>17
      | P+A*BCIN-PCIN
      | P+A*BCIN-PCIN>>17
      | P+A+C
      | P+A+C+CARRYIN
      | P+A+CARRYIN
      | P+A+P
      | P+A+P+CARRYIN
      | P+A+P>>17
      | P+A+P>>17+CARRYIN
      | P+A+PCIN
      | P+A+PCIN+CARRYIN
      | P+A+PCIN>>17
      | P+A+PCIN>>17+CARRYIN
      | P+A-C
      | P+A-P
      | P+A-P>>17
      | P+A-PCIN
      | P+A-PCIN>>17
      | P+ACIN
      | P+ACIN*ACIN
      | P+ACIN*ACIN+C
      | P+ACIN*ACIN+C+CARRYIN
      | P+ACIN*ACIN+CARRYIN
      | P+ACIN*ACIN+P
      | P+ACIN*ACIN+P+CARRYIN
      | P+ACIN*ACIN+P>>17
      | P+ACIN*ACIN+P>>17+CARRYIN
      | P+ACIN*ACIN+PCIN
      | P+ACIN*ACIN+PCIN+CARRYIN
      | P+ACIN*ACIN+PCIN>>17
      | P+ACIN*ACIN+PCIN>>17+CARRYIN
      | P+ACIN*ACIN-C
      | P+ACIN*ACIN-P
      | P+ACIN*ACIN-P>>17
      | P+ACIN*ACIN-PCIN
      | P+ACIN*ACIN-PCIN>>17
      | P+ACIN*B
      | P+ACIN*B+C
      | P+ACIN*B+C+CARRYIN
      | P+ACIN*B+CARRYIN
      | P+ACIN*B+P
      | P+ACIN*B+P+CARRYIN
      | P+ACIN*B+P>>17
      | P+ACIN*B+P>>17+CARRYIN
      | P+ACIN*B+PCIN
      | P+ACIN*B+PCIN+CARRYIN
      | P+ACIN*B+PCIN>>17
      | P+ACIN*B+PCIN>>17+CARRYIN
      | P+ACIN*B-C
      | P+ACIN*B-P
      | P+ACIN*B-P>>17
      | P+ACIN*B-PCIN
      | P+ACIN*B-PCIN>>17
      | P+ACIN*BCIN
      | P+ACIN*BCIN+C
      | P+ACIN*BCIN+C+CARRYIN
      | P+ACIN*BCIN+CARRYIN
      | P+ACIN*BCIN+P
      | P+ACIN*BCIN+P+CARRYIN
      | P+ACIN*BCIN+P>>17
      | P+ACIN*BCIN+P>>17+CARRYIN
      | P+ACIN*BCIN+PCIN
      | P+ACIN*BCIN+PCIN+CARRYIN
      | P+ACIN*BCIN+PCIN>>17
      | P+ACIN*BCIN+PCIN>>17+CARRYIN
      | P+ACIN*BCIN-C
      | P+ACIN*BCIN-P
      | P+ACIN*BCIN-P>>17
      | P+ACIN*BCIN-PCIN
      | P+ACIN*BCIN-PCIN>>17
      | P+ACIN+C
      | P+ACIN+C+CARRYIN
      | P+ACIN+CARRYIN
      | P+ACIN+P
      | P+ACIN+P+CARRYIN
      | P+ACIN+P>>17
      | P+ACIN+P>>17+CARRYIN
      | P+ACIN+PCIN
      | P+ACIN+PCIN+CARRYIN
      | P+ACIN+PCIN>>17
      | P+ACIN+PCIN>>17+CARRYIN
      | P+ACIN-C
      | P+ACIN-P
      | P+ACIN-P>>17
      | P+ACIN-PCIN
      | P+ACIN-PCIN>>17
      | P+B*(A+D)
      | P+B*(A+D)+C
      | P+B*(A+D)+C+CARRYIN
      | P+B*(A+D)+CARRYIN
      | P+B*(A+D)+P
      | P+B*(A+D)+P+CARRYIN
      | P+B*(A+D)+P>>17
      | P+B*(A+D)+P>>17+CARRYIN
      | P+B*(A+D)+PCIN
      | P+B*(A+D)+PCIN+CARRYIN
      | P+B*(A+D)+PCIN>>17
      | P+B*(A+D)+PCIN>>17+CARRYIN
      | P+B*(A+D)-C
      | P+B*(A+D)-P
      | P+B*(A+D)-P>>17
      | P+B*(A+D)-PCIN
      | P+B*(A+D)-PCIN>>17
      | P+B*(ACIN+D)
      | P+B*(ACIN+D)+C
      | P+B*(ACIN+D)+C+CARRYIN
      | P+B*(ACIN+D)+CARRYIN
      | P+B*(ACIN+D)+P
      | P+B*(ACIN+D)+P+CARRYIN
      | P+B*(ACIN+D)+P>>17
      | P+B*(ACIN+D)+P>>17+CARRYIN
      | P+B*(ACIN+D)+PCIN
      | P+B*(ACIN+D)+PCIN+CARRYIN
      | P+B*(ACIN+D)+PCIN>>17
      | P+B*(ACIN+D)+PCIN>>17+CARRYIN
      | P+B*(ACIN+D)-C
      | P+B*(ACIN+D)-P
      | P+B*(ACIN+D)-P>>17
      | P+B*(ACIN+D)-PCIN
      | P+B*(ACIN+D)-PCIN>>17
      | P+B*(D+A)
      | P+B*(D+A)+C
      | P+B*(D+A)+C+CARRYIN
      | P+B*(D+A)+CARRYIN
      | P+B*(D+A)+P
      | P+B*(D+A)+P+CARRYIN
      | P+B*(D+A)+P>>17
      | P+B*(D+A)+P>>17+CARRYIN
      | P+B*(D+A)+PCIN
      | P+B*(D+A)+PCIN+CARRYIN
      | P+B*(D+A)+PCIN>>17
      | P+B*(D+A)+PCIN>>17+CARRYIN
      | P+B*(D+A)-C
      | P+B*(D+A)-P
      | P+B*(D+A)-P>>17
      | P+B*(D+A)-PCIN
      | P+B*(D+A)-PCIN>>17
      | P+B*(D+ACIN)
      | P+B*(D+ACIN)+C
      | P+B*(D+ACIN)+C+CARRYIN
      | P+B*(D+ACIN)+CARRYIN
      | P+B*(D+ACIN)+P
      | P+B*(D+ACIN)+P+CARRYIN
      | P+B*(D+ACIN)+P>>17
      | P+B*(D+ACIN)+P>>17+CARRYIN
      | P+B*(D+ACIN)+PCIN
      | P+B*(D+ACIN)+PCIN+CARRYIN
      | P+B*(D+ACIN)+PCIN>>17
      | P+B*(D+ACIN)+PCIN>>17+CARRYIN
      | P+B*(D+ACIN)-C
      | P+B*(D+ACIN)-P
      | P+B*(D+ACIN)-P>>17
      | P+B*(D+ACIN)-PCIN
      | P+B*(D+ACIN)-PCIN>>17
      | P+B*(D-A)
      | P+B*(D-A)+C
      | P+B*(D-A)+C+CARRYIN
      | P+B*(D-A)+CARRYIN
      | P+B*(D-A)+P
      | P+B*(D-A)+P+CARRYIN
      | P+B*(D-A)+P>>17
      | P+B*(D-A)+P>>17+CARRYIN
      | P+B*(D-A)+PCIN
      | P+B*(D-A)+PCIN+CARRYIN
      | P+B*(D-A)+PCIN>>17
      | P+B*(D-A)+PCIN>>17+CARRYIN
      | P+B*(D-A)-C
      | P+B*(D-A)-P
      | P+B*(D-A)-P>>17
      | P+B*(D-A)-PCIN
      | P+B*(D-A)-PCIN>>17
      | P+B*(D-ACIN)
      | P+B*(D-ACIN)+C
      | P+B*(D-ACIN)+C+CARRYIN
      | P+B*(D-ACIN)+CARRYIN
      | P+B*(D-ACIN)+P
      | P+B*(D-ACIN)+P+CARRYIN
      | P+B*(D-ACIN)+P>>17
      | P+B*(D-ACIN)+P>>17+CARRYIN
      | P+B*(D-ACIN)+PCIN
      | P+B*(D-ACIN)+PCIN+CARRYIN
      | P+B*(D-ACIN)+PCIN>>17
      | P+B*(D-ACIN)+PCIN>>17+CARRYIN
      | P+B*(D-ACIN)-C
      | P+B*(D-ACIN)-P
      | P+B*(D-ACIN)-P>>17
      | P+B*(D-ACIN)-PCIN
      | P+B*(D-ACIN)-PCIN>>17
      | P+B*A
      | P+B*A+C
      | P+B*A+C+CARRYIN
      | P+B*A+CARRYIN
      | P+B*A+P
      | P+B*A+P+CARRYIN
      | P+B*A+P>>17
      | P+B*A+P>>17+CARRYIN
      | P+B*A+PCIN
      | P+B*A+PCIN+CARRYIN
      | P+B*A+PCIN>>17
      | P+B*A+PCIN>>17+CARRYIN
      | P+B*A-C
      | P+B*A-P
      | P+B*A-P>>17
      | P+B*A-PCIN
      | P+B*A-PCIN>>17
      | P+B*ACIN
      | P+B*ACIN+C
      | P+B*ACIN+C+CARRYIN
      | P+B*ACIN+CARRYIN
      | P+B*ACIN+P
      | P+B*ACIN+P+CARRYIN
      | P+B*ACIN+P>>17
      | P+B*ACIN+P>>17+CARRYIN
      | P+B*ACIN+PCIN
      | P+B*ACIN+PCIN+CARRYIN
      | P+B*ACIN+PCIN>>17
      | P+B*ACIN+PCIN>>17+CARRYIN
      | P+B*ACIN-C
      | P+B*ACIN-P
      | P+B*ACIN-P>>17
      | P+B*ACIN-PCIN
      | P+B*ACIN-PCIN>>17
      | P+B*D
      | P+B*D+C
      | P+B*D+C+CARRYIN
      | P+B*D+CARRYIN
      | P+B*D+P
      | P+B*D+P+CARRYIN
      | P+B*D+P>>17
      | P+B*D+P>>17+CARRYIN
      | P+B*D+PCIN
      | P+B*D+PCIN+CARRYIN
      | P+B*D+PCIN>>17
      | P+B*D+PCIN>>17+CARRYIN
      | P+B*D-C
      | P+B*D-P
      | P+B*D-P>>17
      | P+B*D-PCIN
      | P+B*D-PCIN>>17
      | P+BCIN*(A+D)
      | P+BCIN*(A+D)+C
      | P+BCIN*(A+D)+C+CARRYIN
      | P+BCIN*(A+D)+CARRYIN
      | P+BCIN*(A+D)+P
      | P+BCIN*(A+D)+P+CARRYIN
      | P+BCIN*(A+D)+P>>17
      | P+BCIN*(A+D)+P>>17+CARRYIN
      | P+BCIN*(A+D)+PCIN
      | P+BCIN*(A+D)+PCIN+CARRYIN
      | P+BCIN*(A+D)+PCIN>>17
      | P+BCIN*(A+D)+PCIN>>17+CARRYIN
      | P+BCIN*(A+D)-C
      | P+BCIN*(A+D)-P
      | P+BCIN*(A+D)-P>>17
      | P+BCIN*(A+D)-PCIN
      | P+BCIN*(A+D)-PCIN>>17
      | P+BCIN*(ACIN+D)
      | P+BCIN*(ACIN+D)+C
      | P+BCIN*(ACIN+D)+C+CARRYIN
      | P+BCIN*(ACIN+D)+CARRYIN
      | P+BCIN*(ACIN+D)+P
      | P+BCIN*(ACIN+D)+P+CARRYIN
      | P+BCIN*(ACIN+D)+P>>17
      | P+BCIN*(ACIN+D)+P>>17+CARRYIN
      | P+BCIN*(ACIN+D)+PCIN
      | P+BCIN*(ACIN+D)+PCIN+CARRYIN
      | P+BCIN*(ACIN+D)+PCIN>>17
      | P+BCIN*(ACIN+D)+PCIN>>17+CARRYIN
      | P+BCIN*(ACIN+D)-C
      | P+BCIN*(ACIN+D)-P
      | P+BCIN*(ACIN+D)-P>>17
      | P+BCIN*(ACIN+D)-PCIN
      | P+BCIN*(ACIN+D)-PCIN>>17
      | P+BCIN*(D+A)
      | P+BCIN*(D+A)+C
      | P+BCIN*(D+A)+C+CARRYIN
      | P+BCIN*(D+A)+CARRYIN
      | P+BCIN*(D+A)+P
      | P+BCIN*(D+A)+P+CARRYIN
      | P+BCIN*(D+A)+P>>17
      | P+BCIN*(D+A)+P>>17+CARRYIN
      | P+BCIN*(D+A)+PCIN
      | P+BCIN*(D+A)+PCIN+CARRYIN
      | P+BCIN*(D+A)+PCIN>>17
      | P+BCIN*(D+A)+PCIN>>17+CARRYIN
      | P+BCIN*(D+A)-C
      | P+BCIN*(D+A)-P
      | P+BCIN*(D+A)-P>>17
      | P+BCIN*(D+A)-PCIN
      | P+BCIN*(D+A)-PCIN>>17
      | P+BCIN*(D+ACIN)
      | P+BCIN*(D+ACIN)+C
      | P+BCIN*(D+ACIN)+C+CARRYIN
      | P+BCIN*(D+ACIN)+CARRYIN
      | P+BCIN*(D+ACIN)+P
      | P+BCIN*(D+ACIN)+P+CARRYIN
      | P+BCIN*(D+ACIN)+P>>17
      | P+BCIN*(D+ACIN)+P>>17+CARRYIN
      | P+BCIN*(D+ACIN)+PCIN
      | P+BCIN*(D+ACIN)+PCIN+CARRYIN
      | P+BCIN*(D+ACIN)+PCIN>>17
      | P+BCIN*(D+ACIN)+PCIN>>17+CARRYIN
      | P+BCIN*(D+ACIN)-C
      | P+BCIN*(D+ACIN)-P
      | P+BCIN*(D+ACIN)-P>>17
      | P+BCIN*(D+ACIN)-PCIN
      | P+BCIN*(D+ACIN)-PCIN>>17
      | P+BCIN*(D-A)
      | P+BCIN*(D-A)+C
      | P+BCIN*(D-A)+C+CARRYIN
      | P+BCIN*(D-A)+CARRYIN
      | P+BCIN*(D-A)+P
      | P+BCIN*(D-A)+P+CARRYIN
      | P+BCIN*(D-A)+P>>17
      | P+BCIN*(D-A)+P>>17+CARRYIN
      | P+BCIN*(D-A)+PCIN
      | P+BCIN*(D-A)+PCIN+CARRYIN
      | P+BCIN*(D-A)+PCIN>>17
      | P+BCIN*(D-A)+PCIN>>17+CARRYIN
      | P+BCIN*(D-A)-C
      | P+BCIN*(D-A)-P
      | P+BCIN*(D-A)-P>>17
      | P+BCIN*(D-A)-PCIN
      | P+BCIN*(D-A)-PCIN>>17
      | P+BCIN*(D-ACIN)
      | P+BCIN*(D-ACIN)+C
      | P+BCIN*(D-ACIN)+C+CARRYIN
      | P+BCIN*(D-ACIN)+CARRYIN
      | P+BCIN*(D-ACIN)+P
      | P+BCIN*(D-ACIN)+P+CARRYIN
      | P+BCIN*(D-ACIN)+P>>17
      | P+BCIN*(D-ACIN)+P>>17+CARRYIN
      | P+BCIN*(D-ACIN)+PCIN
      | P+BCIN*(D-ACIN)+PCIN+CARRYIN
      | P+BCIN*(D-ACIN)+PCIN>>17
      | P+BCIN*(D-ACIN)+PCIN>>17+CARRYIN
      | P+BCIN*(D-ACIN)-C
      | P+BCIN*(D-ACIN)-P
      | P+BCIN*(D-ACIN)-P>>17
      | P+BCIN*(D-ACIN)-PCIN
      | P+BCIN*(D-ACIN)-PCIN>>17
      | P+BCIN*A
      | P+BCIN*A+C
      | P+BCIN*A+C+CARRYIN
      | P+BCIN*A+CARRYIN
      | P+BCIN*A+P
      | P+BCIN*A+P+CARRYIN
      | P+BCIN*A+P>>17
      | P+BCIN*A+P>>17+CARRYIN
      | P+BCIN*A+PCIN
      | P+BCIN*A+PCIN+CARRYIN
      | P+BCIN*A+PCIN>>17
      | P+BCIN*A+PCIN>>17+CARRYIN
      | P+BCIN*A-C
      | P+BCIN*A-P
      | P+BCIN*A-P>>17
      | P+BCIN*A-PCIN
      | P+BCIN*A-PCIN>>17
      | P+BCIN*ACIN
      | P+BCIN*ACIN+C
      | P+BCIN*ACIN+C+CARRYIN
      | P+BCIN*ACIN+CARRYIN
      | P+BCIN*ACIN+P
      | P+BCIN*ACIN+P+CARRYIN
      | P+BCIN*ACIN+P>>17
      | P+BCIN*ACIN+P>>17+CARRYIN
      | P+BCIN*ACIN+PCIN
      | P+BCIN*ACIN+PCIN+CARRYIN
      | P+BCIN*ACIN+PCIN>>17
      | P+BCIN*ACIN+PCIN>>17+CARRYIN
      | P+BCIN*ACIN-C
      | P+BCIN*ACIN-P
      | P+BCIN*ACIN-P>>17
      | P+BCIN*ACIN-PCIN
      | P+BCIN*ACIN-PCIN>>17
      | P+BCIN*D
      | P+BCIN*D+C
      | P+BCIN*D+C+CARRYIN
      | P+BCIN*D+CARRYIN
      | P+BCIN*D+P
      | P+BCIN*D+P+CARRYIN
      | P+BCIN*D+P>>17
      | P+BCIN*D+P>>17+CARRYIN
      | P+BCIN*D+PCIN
      | P+BCIN*D+PCIN+CARRYIN
      | P+BCIN*D+PCIN>>17
      | P+BCIN*D+PCIN>>17+CARRYIN
      | P+BCIN*D-C
      | P+BCIN*D-P
      | P+BCIN*D-P>>17
      | P+BCIN*D-PCIN
      | P+BCIN*D-PCIN>>17
      | P+C
      | P+C+(A+D)
      | P+C+(A+D)*(A+D)
      | P+C+(A+D)*(A+D)+CARRYIN
      | P+C+(A+D)*B
      | P+C+(A+D)*B+CARRYIN
      | P+C+(A+D)*BCIN
      | P+C+(A+D)*BCIN+CARRYIN
      | P+C+(A+D)+CARRYIN
      | P+C+(ACIN+D)
      | P+C+(ACIN+D)*(ACIN+D)
      | P+C+(ACIN+D)*(ACIN+D)+CARRYIN
      | P+C+(ACIN+D)*B
      | P+C+(ACIN+D)*B+CARRYIN
      | P+C+(ACIN+D)*BCIN
      | P+C+(ACIN+D)*BCIN+CARRYIN
      | P+C+(ACIN+D)+CARRYIN
      | P+C+(D+A)
      | P+C+(D+A)*(D+A)
      | P+C+(D+A)*(D+A)+CARRYIN
      | P+C+(D+A)*B
      | P+C+(D+A)*B+CARRYIN
      | P+C+(D+A)*BCIN
      | P+C+(D+A)*BCIN+CARRYIN
      | P+C+(D+A)+CARRYIN
      | P+C+(D+ACIN)
      | P+C+(D+ACIN)*(D+ACIN)
      | P+C+(D+ACIN)*(D+ACIN)+CARRYIN
      | P+C+(D+ACIN)*B
      | P+C+(D+ACIN)*B+CARRYIN
      | P+C+(D+ACIN)*BCIN